simulink model "vegas01_4tap_1024ch_220_r8b_opt"  (Simulink 7.5) (continued)
== system vegas01_4tap_1024ch_220_r8b_opt == (continued)
      | input_bit_width = eval(get_param(gcb,'input_bit_width'));
      | if input_bit_width + bit_growth_sum > 18
      |     disp('Total bit width cannot exceed 18. Forcing to 0 bit growth');
      |     set_param(gcb,'bit_growth_chart','[0]');
      | end|||||||||||fft_callback_arch(gcb);||||specify_mult = get_param(gcb, 'specify_mult');
      | set_param_state(gcb, 'mult_spec', specify_mult);||hardcode_shifts = get_param(gcb, 'hardcode_shifts');
      | set_param_state(gcb, 'shift_schedule', hardcode_shifts);||fft_callback_dsp48_adders(gcb);|bit_growth_chart = eval(get_param(gcb,'bit_growth_chart'));
      | FFTSize = eval(get_param(gcb,'FFTSize'));
      | bit_growth_chart = [reshape(bit_growth_chart, 1, []) zeros(1,FFTSize)];
      | bit_growth_sum = sum(bit_growth_chart(1:FFTSize));
      | input_bit_width = eval(get_param(gcb,'input_bit_width'));
      | if input_bit_width + bit_growth_sum > 18
      |     disp('Total bit width cannot exceed 18. Forcing to 0 bit growth');
      |     set_param(gcb,'bit_growth_chart','[0]');
      | end||
      mask TabNameString: Basic,Basic,Basic,Basic,Basic,Latency,Latency,Latency,Latency,Latency,Latency,Implementation,Implementation,Implementation,Implementation,Implementation,Implementation,Implementation,Implementation,Implementation,Implementation,Implementation,Basic,Basic,Basic
  gbe0 : xps_library/ten_Gbe_v2  Tag=xps:tengbe_v2  SystemSampleTime=-1  port=0  rx_dist_ram=on  large_frames=on  show_param=on  pre_emph=3  swing=800  fab_en=off  fab_mac=hex2dec('123456780000')  fab_ip=10*(2^24) + 0*(2^16) + 0*(2^8) + 140*(2^0)  fab_udp=60001  fab_gate=1  cpu_rx_en=on  cpu_tx_en=on
  gpio : xps_library/gpio  Tag=xps:gpio  SystemSampleTime=-1  io_group=ROACH:gpiob  io_dir=in  arith_type=Unsigned  bitwidth=4  bin_pt=0  bit_index=[1:4]  sample_period=1  use_single_ended=off  use_ddr=off  reg_iob=on  reg_clk_phase=0  termination=None
      link override ssg_speader_r2_gpio_gateway: arith_type=Unsigned  n_bits=4  bin_pt=0  period=sample_period
  gpio_tig_reg_1 : xbsIndex_r4/Register  init=0  rst=off  en=off  dbl_ovrd=off  block_type=register
      settings: block_version=11.4
  gpio_tig_reg_2 : xbsIndex_r4/Register  init=0  rst=off  en=off  dbl_ovrd=off  block_type=register
      settings: block_version=11.4
  gpio_tig_reg_3 : xbsIndex_r4/Register  init=0  rst=off  en=off  dbl_ovrd=off  block_type=register
      settings: block_version=11.4
  leds_gpios : Subsystem
  length : xps_library/software register  Tag=xps:sw_reg  SystemSampleTime=-1  io_dir=From Processor  arith_type=Unsigned  bitwidth=32  bin_pt=0  sample_period=1  gw_name=vegas01_4tap_1024ch_220_r8b_length_user_data_out
      link override ssg_speader_r2_length_user_data_out: arith_type=Unsigned  n_bits=32  bin_pt=0  period=1
  manrst : xps_library/software register  Tag=xps:sw_reg  SystemSampleTime=-1  io_dir=From Processor  arith_type=Unsigned  bitwidth=32  bin_pt=0  sample_period=1  gw_name=vegas01_4tap_1024ch_220_r8b_manrst_user_data_out
      link override convert: arith_type=Unsigned  n_bits=32  bin_pt=0
      link override r_spec_2048_r102_cnt_rst_user_data_out: arith_type=Unsigned  n_bits=32  bin_pt=0  period=1
      link override r_spec_2048_r102_nosim_cnt_rst_user_data_out: arith_type=Unsigned  n_bits=32  bin_pt=0  period=1
      link override r_spec_2048_r103_cnt_rst_user_data_out: arith_type=Unsigned  n_bits=32  bin_pt=0  period=1
      link override roach_spec_no10gbe_sync_cnt_rst_user_data_out: arith_type=Unsigned  n_bits=32  bin_pt=0  period=1
      link override roach_spec_rst_cnt_user_data_out: arith_type=Unsigned  n_bits=32  bin_pt=0  period=1
      link override roach_spec_rst_sync_cnt_user_data_out: arith_type=Unsigned  n_bits=32  bin_pt=0  period=1
  master_slave_sel : xps_library/software register  Tag=xps:sw_reg  SystemSampleTime=-1  io_dir=From Processor  arith_type=Unsigned  bitwidth=32  bin_pt=0  sample_period=1  gw_name=vegas01_4tap_1024ch_220_r8b_master_slave_sel_user_data_out
      link override ssg_speader_r2_master_slave_sel_user_data_out: arith_type=Unsigned  n_bits=32  bin_pt=0  period=1
  negedge : casper_library_misc/negedge  Tag=Rising Edge Detector  SystemSampleTime=-1
  negedge1 : casper_library_misc/negedge  Tag=Rising Edge Detector  SystemSampleTime=-1
  negedge2 : casper_library_misc/negedge  Tag=Rising Edge Detector  SystemSampleTime=-1
  pfb_fir_real1 : Subsystem  mask(pfb_coeff_gen_dual){pfb_size=11, n_sim_inputs=3, n_taps=4, data_n_bits=8, coeff_n_bits=18, output_bit_width=18, window_fn=hamming, bin_width=1, end_scale_val=1, endDelay=on, cheap_sync=off, autoplace_mode='Attached to ADC0', autoplace_optimize=off, bram_latency_coeff=off, bram_latency_delay=off, register_counter=on, pack_delays=on}
      mask prompts: pfb_size "PFB Size (2^?)"; n_sim_inputs "# Simultaneous Inputs (2^?); Max=6"; n_taps "# Taps"; data_n_bits "Input bit Width"; coeff_n_bits "# Coefficient Bits"; output_bit_width "Output bit width"; window_fn "Window Function"; bin_width "Channel Width"; end_scale_val "Downscale at end (2^?)"; endDelay "Delay at end?"; cheap_sync "Use cheap Sync hardware?"; autoplace_mode "Autoplacement Mode?"; autoplace_optimize "Optimize parameters for auto-placement? (settings below here ignored)"; bram_latency_coeff "Register Coefficient Brams?"; bram_latency_delay "Register Delay Brams?"; register_counter "Register Delay Counters? (0 or 1)"; pack_delays "Pack delays cross-input?"
      mask init:
      | global drawing_parameters;
      | % drawing_parameters.pfb_size=pfb_size;
      | % drawing_parameters.n_sim_inputs=n_sim_inputs;
      | % drawing_parameters.n_taps=n_taps;
      | % drawing_parameters.data_n_bits=data_n_bits;
      | % drawing_parameters.data_bin_pt=data_bin_pt;
      | % drawing_parameters.coeff_n_bits=coeff_n_bits;
      | % drawing_parameters.coeff_bin_pt=coeff_n_bits-1;
      | % drawing_parameters.window_fn=window_fn;
      | % drawing_parameters.mem_type=mem_type;
      | % drawing_parameters.bram_latency=bram_latency;
      | % drawing_parameters.bin_width=bin_width;
      | % drawing_parameters.end_scale_val=end_scale_val;
      | % drawing_parameters.cheap_sync=cheap_sync;
      | config.source=str2func('pfb_improved_draw');
      | config.toplevel=gcb;
      | config.debug=0;
      | config.depend={'pfb_improved_draw.m','pfb_coeff_gen_dual_draw.m'};
      | xBlock(config,{pfb_size,n_sim_inputs,n_taps,data_n_bits,coeff_n_bits, ...
      |                output_bit_width,window_fn,bram_latency_coeff+2,bram_latency_delay+2,bin_width,end_scale_val,endDelay, cheap_sync,register_counter, pack_delays, autoplace_mode, autoplace_optimize});
      mask StyleString: edit,edit,edit,edit,edit,edit,popup(bartlett|barthannwin|blackman|blackmanharris|bohamwin|chebwin|flattopwin|gausswin|hamming|hann|kaiser|nuttallwin|parzenwin|rectwin|tukeywin|triang),edit,edit,checkbox,checkbox,popup('Attached to ADC0'|'Attached to ADC1'|'Attached to both ADC0 and ADC1 (interleaved)'),checkbox,checkbox,checkbox,checkbox,checkbox
  pfb_fir_real3 : Subsystem  mask(pfb_coeff_gen_dual){pfb_size=11, n_sim_inputs=3, n_taps=4, data_n_bits=8, coeff_n_bits=18, output_bit_width=18, window_fn=hamming, bin_width=1, end_scale_val=1, endDelay=on, cheap_sync=off, autoplace_mode='Attached to ADC0', autoplace_optimize=off, bram_latency_coeff=off, bram_latency_delay=off, register_counter=on, pack_delays=on}
      mask prompts: pfb_size "PFB Size (2^?)"; n_sim_inputs "# Simultaneous Inputs (2^?); Max=6"; n_taps "# Taps"; data_n_bits "Input bit Width"; coeff_n_bits "# Coefficient Bits"; output_bit_width "Output bit width"; window_fn "Window Function"; bin_width "Channel Width"; end_scale_val "Downscale at end (2^?)"; endDelay "Delay at end?"; cheap_sync "Use cheap Sync hardware?"; autoplace_mode "Autoplacement Mode?"; autoplace_optimize "Optimize parameters for auto-placement? (settings below here ignored)"; bram_latency_coeff "Register Coefficient Brams?"; bram_latency_delay "Register Delay Brams?"; register_counter "Register Delay Counters? (0 or 1)"; pack_delays "Pack delays cross-input?"
      mask init: same as vegas01_4tap_1024ch_220_r8b_opt/pfb_fir_real1
      mask StyleString: edit,edit,edit,edit,edit,edit,popup(bartlett|barthannwin|blackman|blackmanharris|bohamwin|chebwin|flattopwin|gausswin|hamming|hann|kaiser|nuttallwin|parzenwin|rectwin|tukeywin|triang),edit,edit,checkbox,checkbox,popup('Attached to ADC0'|'Attached to ADC1'|'Attached to both ADC0 and ADC1 (interleaved)'),checkbox,checkbox,checkbox,checkbox,checkbox
  posedge1 : casper_library_misc/posedge  Tag=Rising Edge Detector  SystemSampleTime=-1
  posedge3 : casper_library_misc/posedge  Tag=Rising Edge Detector  SystemSampleTime=-1
  posedge7 : casper_library_misc/posedge  Tag=Rising Edge Detector  SystemSampleTime=-1
  reinterp_vacc{,1..15} (16 alike) : xbsIndex_r4/Reinterpret  force_arith_type=on  arith_type="Signed  (2's comp)"  force_bin_pt=off  bin_pt=0  block_type=reinterpret
  reshaper : Subsystem  mask{inwid=512, inlen=256, outwid=64, outchunk=1024, headlen=2000}
      mask prompts: inwid "Input Width (bits)"; inlen "Input Length (clocks)"; outwid "Output Width (bits)"; outchunk "Output Chunk Length (clocks)"; headlen "Header Length"
      mask help:
      | This block takes wide input data and reshapes it to a narrower (or the same)
      | bit width, and breaks it into packets, between which a header can be inserted.
      | Each input data frame must be proceeded by a reset pulse. The input data can be
      | disjoint, with a pulsed valid signal. When the reset signal is pulsed, the previous data
      | frame will be read out. Therefore the number of clocks to read out the header and
      | data must be greater than the number of clocks between two frames (rst signals).
      |
      | The sel signal can be used to drive the select input of a mux which muxes between
      | the data stream (dout) and the indicated number of header words. The header_valid_out
      | signal goes high for each word of the header. dout should be connected to input
      | 0 of the mux. The data_valid_out goes high for each output data value. The st output
      | is for debug access to the internal state machine state.
      mask init:
      | l2inlen = ceil(log2(inlen))
      | l2outchunk = ceil(log2(outchunk))
      | outlen = inlen*(inwid/outwid)
      | nchunk = outlen/outchunk
      | l2headlen = ceil(log2(headlen))
      | l2outlen = ceil(log2(outlen))
  sg : Subsystem
  speader1 : Subsystem  AncestorBlock=gbt_library/speader  mask{spead_msw=64, spead_lsw=40, num_items=6, num_payloads=2}
      mask prompts: num_items "Number of items (+5)"; num_payloads "number of payloads"
      mask help:
      | The size of the heap and payload are in Bytes.
      | If there is one packet per heap then payload_offset is 0.
      | All the input must be not longer than 40 bits.
  spectrum_cntr : xbsIndex_r4/Counter  cnt_type=Free Running  cnt_to=Inf  operation=Up  start_count=0  cnt_by_val=1  arith_type=Unsigned  n_bits=40  bin_pt=0  load_pin=off  rst=on  en=on  explicit_period=on  period=1  dbl_ovrd=off  use_behavioral_HDL=off  implementation=Fabric  block_type=counter
      settings: block_version=11.4
  ssg_bram : Subsystem  Tag=xps:bram  AncestorBlock=xps_library/Shared BRAM  mask{arith_type=Unsigned, addr_width=10, data_width=32, data_bin_pt=0, init_vals=[12801,121600,3201,3203,57602,3203,3201,121600,12801,12805,121604,3205,3207,57606,3207,3205,121604,12805,0:0], sample_rate=1}
      mask prompts: arith_type "Output Data Type"; addr_width "Address width"; data_width "Data Width"; data_bin_pt "Data Binary Point"; init_vals "Initial 32-bit values (simulation only)"; sample_rate "Sample rate"
      mask init:
      | shared_bram_mask;
      mask StyleString: popup(Unsigned|Signed  (2's comp)),edit,popup(8|16|32|64|128),edit,edit,edit
  ssg_bram_delay1 : xbsIndex_r4/Delay  rst=off  infoeditControl=Selection of Reset will increase slice count due to use of real FFs and instead of SRLs  en=off  latency=1  dbl_ovrd=off  reg_retiming=on  block_type=delay
  ssg_bram_delay2 : xbsIndex_r4/Delay  rst=off  infoeditControl=Selection of Reset will increase slice count due to use of real FFs and instead of SRLs  en=off  latency=1  dbl_ovrd=off  reg_retiming=off  block_type=delay
  stokes : Subsystem  mask{n_samples=4, addlatency=2}
      mask prompts: n_samples "Number of Simultaneous Inputs"; addlatency "Add Latency"
      mask init:
      | % % To make the xBlock smart so it won''t re-generate the
      | % % subsystem if neither the arguments nor the scripts are
      | % % changes, use as the following:
      | % cfg.source = str2func('stokes_init');
      | % cfg.toplevel = gcb;
      | % cfg.depend = {'stokes_init'};
      | % args = {n_samples, addlatency};
      | % xBlock(cfg, args);
      mask TabNameString: Basic,Basic
  time_cntr : xbsIndex_r4/Counter  cnt_type=Free Running  cnt_to=Inf  operation=Up  start_count=0  cnt_by_val=1  arith_type=Unsigned  n_bits=40  bin_pt=0  load_pin=off  rst=on  en=off  explicit_period=on  period=1  dbl_ovrd=off  use_behavioral_HDL=off  implementation=Fabric  block_type=counter
      settings: block_version=11.4
  top bit : xbsIndex_r4/Slice  nbits=1  boolean_output=on  mode=Upper Bit Location + Width  bit1=0  base1=MSB of Input  bit0=0  base0=LSB of Input  dbl_ovrd=off  block_type=slice
  vacc0 : Subsystem  AncestorBlock=gavrt_library/vacc  mask(vacc){veclen=8, n_inputs=4, max_accum=10, arith_type=1, in_bit_width=32, in_bin_pt=0, out_bit_width=42, out_bin_pt=0, register_din=1, add_latency=2, bram_latency=2, mux_latency=1, use_dsp48=1}
      mask prompts: veclen "Vector Length (2^?)"; n_inputs "Number of Simultaneous Vectors"; max_accum "Maximum # Accumulations (2^?)"; arith_type "Arithmetic Type (0=Unsigned, 1=Signed)"; in_bit_width "Input Bit Width"; in_bin_pt "Input Binary Point"; out_bit_width "Output Bit Width"; out_bin_pt "Output Binary Point"; register_din "Register Input Data  (1 = Enable Register Retiming on input delay)"; add_latency "Add Latency"; bram_latency "BRAM Latency"; mux_latency "Mux Latency"; use_dsp48 "Use DSP48"
      mask help:
      | Sync is expected the clock before the first data to be accumulated.
      | Use the Cram block to feed din for multiple simultaneous inputs.
      | acc_len is the number of vectors to sum.
      | valid is high while a valid accumulation is being clocked out on dout.
      | If only one sync pulse is provided, valid accumulations will repeatedly appear on dout as they become available.
      | Undesired behavior may occur if acc_len is changed without a new sync pulse.
      | Note: The output is an unsigned concatenation of the output streams. Thus, even with only one stream, the output must be recast before using.
      | Register Input Data controls the "Enable Register Retiming" parameter of the delays on 'din'.
      mask init:
      | config.toplevel = gcb;
      | config.source = str2func('vacc_init_xblock');
      | xBlock( config, {...
      |     'veclen', veclen, ...
      |     'n_inputs', n_inputs, ...
      |     'max_accum', max_accum, ...
      |     'arith_type', arith_type, ...
      |     'in_bit_width', in_bit_width, ...
      |     'in_bin_pt', in_bin_pt, ...
      |     'out_bit_width', out_bit_width, ...
      |     'out_bin_pt', out_bin_pt, ...
      |     'register_din', register_din, ...
      |     'add_latency', add_latency, ...
      |     'bram_latency', bram_latency, ...
      |     'mux_latency', mux_latency, ...
      |     'use_dsp48', use_dsp48, ...
      | });
      settings: AttributesFormatString=|
      | vector length=32, inputs=4
      | max accumulations=2^5
  vacc1 : Subsystem  AncestorBlock=gavrt_library/vacc  mask(vacc){veclen=8, n_inputs=4, max_accum=10, arith_type=1, in_bit_width=32, in_bin_pt=0, out_bit_width=42, out_bin_pt=0, register_din=1, add_latency=2, bram_latency=2, mux_latency=1, use_dsp48=1}
      mask prompts: veclen "Vector Length (2^?)"; n_inputs "Number of Simultaneous Vectors"; max_accum "Maximum # Accumulations (2^?)"; arith_type "Arithmetic Type (0=Unsigned, 1=Signed)"; in_bit_width "Input Bit Width"; in_bin_pt "Input Binary Point"; out_bit_width "Output Bit Width"; out_bin_pt "Output Binary Point"; register_din "Register Input Data  (1 = Enable Register Retiming on input delay)"; add_latency "Add Latency"; bram_latency "BRAM Latency"; mux_latency "Mux Latency"; use_dsp48 "Use DSP48"
      mask help:
      | Sync is expected the clock before the first data to be accumulated.
      | Use the Cram block to feed din for multiple simultaneous inputs.
      | acc_len is the number of vectors to sum.
      | valid is high while a valid accumulation is being clocked out on dout.
      | If only one sync pulse is provided, valid accumulations will repeatedly appear on dout as they become available.
      | Undesired behavior may occur if acc_len is changed without a new sync pulse.
      | Note: The output is an unsigned concatenation of the output streams. Thus, even with only one stream, the output must be recast before using.
      | Register Input Data controls the "Enable Register Retiming" parameter of the delays on 'din'.
      mask init: same as vegas01_4tap_1024ch_220_r8b_opt/vacc0
      settings: AttributesFormatString=|
      | vector length=32, inputs=4
      | max accumulations=2^5
  vacc2 : Subsystem  AncestorBlock=gavrt_library/vacc  mask(vacc){veclen=8, n_inputs=4, max_accum=10, arith_type=1, in_bit_width=32, in_bin_pt=0, out_bit_width=42, out_bin_pt=0, register_din=1, add_latency=2, bram_latency=2, mux_latency=1, use_dsp48=1}
      mask prompts: veclen "Vector Length (2^?)"; n_inputs "Number of Simultaneous Vectors"; max_accum "Maximum # Accumulations (2^?)"; arith_type "Arithmetic Type (0=Unsigned, 1=Signed)"; in_bit_width "Input Bit Width"; in_bin_pt "Input Binary Point"; out_bit_width "Output Bit Width"; out_bin_pt "Output Binary Point"; register_din "Register Input Data  (1 = Enable Register Retiming on input delay)"; add_latency "Add Latency"; bram_latency "BRAM Latency"; mux_latency "Mux Latency"; use_dsp48 "Use DSP48"
      mask help:
      | Sync is expected the clock before the first data to be accumulated.
      | Use the Cram block to feed din for multiple simultaneous inputs.
      | acc_len is the number of vectors to sum.
      | valid is high while a valid accumulation is being clocked out on dout.
      | If only one sync pulse is provided, valid accumulations will repeatedly appear on dout as they become available.
      | Undesired behavior may occur if acc_len is changed without a new sync pulse.
      | Note: The output is an unsigned concatenation of the output streams. Thus, even with only one stream, the output must be recast before using.
      | Register Input Data controls the "Enable Register Retiming" parameter of the delays on 'din'.
      mask init: same as vegas01_4tap_1024ch_220_r8b_opt/vacc0
      settings: AttributesFormatString=|
      | vector length=32, inputs=4
      | max accumulations=2^5
  vacc3 : Subsystem  AncestorBlock=gavrt_library/vacc  mask(vacc){veclen=8, n_inputs=4, max_accum=10, arith_type=1, in_bit_width=32, in_bin_pt=0, out_bit_width=42, out_bin_pt=0, register_din=1, add_latency=2, bram_latency=2, mux_latency=1, use_dsp48=1}
      mask prompts: veclen "Vector Length (2^?)"; n_inputs "Number of Simultaneous Vectors"; max_accum "Maximum # Accumulations (2^?)"; arith_type "Arithmetic Type (0=Unsigned, 1=Signed)"; in_bit_width "Input Bit Width"; in_bin_pt "Input Binary Point"; out_bit_width "Output Bit Width"; out_bin_pt "Output Binary Point"; register_din "Register Input Data  (1 = Enable Register Retiming on input delay)"; add_latency "Add Latency"; bram_latency "BRAM Latency"; mux_latency "Mux Latency"; use_dsp48 "Use DSP48"
      mask help:
      | Sync is expected the clock before the first data to be accumulated.
      | Use the Cram block to feed din for multiple simultaneous inputs.
      | acc_len is the number of vectors to sum.
      | valid is high while a valid accumulation is being clocked out on dout.
      | If only one sync pulse is provided, valid accumulations will repeatedly appear on dout as they become available.
      | Undesired behavior may occur if acc_len is changed without a new sync pulse.
      | Note: The output is an unsigned concatenation of the output streams. Thus, even with only one stream, the output must be recast before using.
      | Register Input Data controls the "Enable Register Retiming" parameter of the delays on 'din'.
      mask init: same as vegas01_4tap_1024ch_220_r8b_opt/vacc0
      settings: AttributesFormatString=|
      | vector length=32, inputs=4
      | max accumulations=2^5
  wires:
  manrst/1 -> Register16/1
  Constant19/1 -> manrst/1
  Register16/1 -> Slice2/1, Slice4/1
  Slice2/1 -> Delay26/1
  Register10/1 -> [xout0]
  Register11/1 -> [xout1]
  Register9/1 -> Delay2/1
  Register48/1 -> [yout0]
  Register49/1 -> [yout1]
  Constant4/1 -> adc083000x2/4(adc1_data_valid_sim), adc083000x2/3(adc1_sync_sim), adc083000x2/2(adc0_data_valid_sim)
  Constant1/1 -> adc083000x2/1(adc0_sync_sim)
  Slice10/1 -> [adc_sync]
  Register44/1 -> Slice10/1
  adc083000x2/1(adc0_s0) -> Delay7/1
  adc083000x2/9(adc0_sync) -> Register44/1
  adc083000x2/10(adc0_outofrange) -> Terminator44/1
  adc083000x2/11(adc0_data_valid) -> Terminator45/1
  adc083000x2/12(adc1_s0) -> Delay27/1
  adc083000x2/20(adc1_sync) -> Terminator43/1
  adc083000x2/21(adc1_outofrange) -> Terminator46/1
  adc083000x2/22(adc1_data_valid) -> Terminator47/1
  Constant20/1 -> adc083000x2/5(sim_adc0_data_in)
  Constant21/1 -> adc083000x2/6(sim_adc1_data_in)
  Concat1/1 -> [default_ip]
  Constant6/1 -> gbe0/7
  gbe0/10 -> Terminator/1
  gbe0/2 -> Terminator17/1
  gbe0/3 -> Terminator16/1
  gbe0/4 -> Terminator15/1
  gbe0/5 -> [txack]
  gbe0/6 -> Terminator8/1
  gbe0/7 -> Terminator6/1
  gbe0/8 -> Terminator5/1
  gbe0/9 -> Terminator3/1
  gbe0/11 -> Terminator11/1
  Constant5/1 -> gbe0/8
  Delay21/1 -> gbe0/6
  Delay25/1 -> gbe0/3
  Delay29/1 -> gbe0/2
  Delay30/1 -> gbe0/1
  acc_len/1 -> Delay32/1
  Constant3/1 -> acc_len/1
  Delay32/1 -> [acc_len]
  Constant2/1 -> [fftshift]
  adc083000x2/16(adc1_s4) -> Delay34/1
  adc083000x2/13(adc1_s1) -> Delay28/1
  adc083000x2/15(adc1_s3) -> Delay33/1
  adc083000x2/17(adc1_s5) -> Delay35/1
  adc083000x2/19(adc1_s7) -> Delay37/1
  adc083000x2/3(adc0_s2) -> Delay18/1
  adc083000x2/5(adc0_s4) -> Delay20/1
  adc083000x2/7(adc0_s6) -> Delay23/1
  adc083000x2/2(adc0_s1) -> Delay9/1
  adc083000x2/4(adc0_s3) -> Delay19/1
  adc083000x2/6(adc0_s5) -> Delay22/1
  adc083000x2/8(adc0_s7) -> Delay24/1
  adc083000x2/14(adc1_s2) -> Delay31/1
  adc083000x2/18(adc1_s6) -> Delay36/1
  err_clear_sim1/1 -> dest_ip/1
  dest_ip/1 -> [dest_ip]
  dest_port/1 -> Slice7/1
  err_clear_sim3/1 -> dest_port/1
  Slice7/1 -> [dest_port]
  Constant/1 -> Concat1/1
  Constant7/1 -> Concat1/2
  Constant8/1 -> Concat1/3
  Constant9/1 -> Concat1/4
  [tge_rst] -> Delay30/1
  [data_out] -> speader1/1(data_in)
  [data_valid] -> speader1/2(valid_in)
  [dest_ip] -> gbe0/4
  [dest_port] -> gbe0/5
  speader1/3(eof) -> [eof]
  [eof] -> Delay21/1
  Constant18/1 -> speader1/3(heap_size)
  Constant33/1 -> speader1/8(mode)
  Constant34/1 -> speader1/10(payload_offset)
  speader1/2(data_valid) -> [data_valid_spead]
  [data_valid_spead] -> Delay25/1
  [data_spead] -> Delay29/1
  [master_rst1] -> time_cntr/1
  Slice5/1 -> [xxout0]
  Slice6/1 -> [yyout0]
  Slice13/1 -> [xyoutre0]
  Slice18/1 -> [xyoutim0]
  Slice19/1 -> [xxout1]
  Slice20/1 -> [yyout1]
  Slice21/1 -> [xyoutre1]
  Slice22/1 -> [xyoutim1]
  [acc_len] -> Convert/1
  Constant24/1 -> speader1/4(Packet_length)
  [master_rst1] -> spectrum_cntr/1
  [eoh] -> spectrum_cntr/2
  [spead_rst] -> speader1/11(Reset)
  Convert/1 -> speader1/7(integration size)
  speader1/1(data_out) -> [data_spead]
  fft_wideband_real1/1(sync_out) -> Register9/1
  [sync_gen_1] -> pfb_fir_real1/1(sync)
  [sync_gen_0] -> pfb_fir_real3/1(sync)
  [a0] -> pfb_fir_real1/2(in0)
  [a1] -> pfb_fir_real1/3(in1)
  [a2] -> pfb_fir_real1/4(in2)
  [a3] -> pfb_fir_real1/5(in3)
  [a4] -> pfb_fir_real1/6(in4)
  [a5] -> pfb_fir_real1/7(in5)
  [a6] -> pfb_fir_real1/8(in6)
  [a7] -> pfb_fir_real1/9(in7)
  [b0] -> pfb_fir_real3/2(in0)
  [b1] -> pfb_fir_real3/3(in1)
  [b2] -> pfb_fir_real3/4(in2)
  [b3] -> pfb_fir_real3/5(in3)
  [b4] -> pfb_fir_real3/6(in4)
  [b5] -> pfb_fir_real3/7(in5)
  [b6] -> pfb_fir_real3/8(in6)
  [b7] -> pfb_fir_real3/9(in7)
  [fftshift] -> Delay5/1
  Delay6/1 -> fft_wideband_real/2(shift)
  Register1/1 -> [xout2]
  Register2/1 -> [xout3]
  fft_wideband_real/1(sync_out) -> Terminator2/1
  Register12/1 -> [yout2]
  Register13/1 -> [yout3]
  [xout0] -> stokes/1(x0)
  [yout0] -> stokes/2(y0)
  [xout1] -> stokes/3(x1)
  [yout1] -> stokes/4(y1)
  [xout2] -> stokes/5(x2)
  [yout2] -> stokes/6(y2)
  [xout3] -> stokes/7(x3)
  [yout3] -> stokes/8(y3)
  Slice29/1 -> [xxout2]
  Slice30/1 -> [yyout2]
  Slice14/1 -> [xyoutre2]
  Slice15/1 -> [xyoutim2]
  Slice25/1 -> [xxout3]
  Slice26/1 -> [yyout3]
  Slice27/1 -> [xyoutre3]
  Slice28/1 -> [xyoutim3]
  stokes/1(x0x0*) -> Slice5/1
  stokes/2(y0y0*) -> Slice6/1
  stokes/3(x0y0*_re) -> Slice13/1
  stokes/4(x0y0*_im) -> Slice18/1
  stokes/5(x1x1*) -> Slice19/1
  stokes/6(y1y1*) -> Slice20/1
  stokes/7(x1y1*_re) -> Slice21/1
  stokes/8(x1y1*_im) -> Slice22/1
  stokes/9(x2x2*) -> Slice29/1
  stokes/10(y2y2*) -> Slice30/1
  stokes/11(x2y2*_re) -> Slice14/1
  stokes/12(x2y2*_im) -> Slice15/1
  stokes/13(x3x3*) -> Slice25/1
  stokes/14(y3y3*) -> Slice26/1
  stokes/15(x3y3*_re) -> Slice27/1
  stokes/16(x3y3*_im) -> Slice28/1
  spectrum_cntr/1 -> [spec_cntr]
  time_cntr/1 -> [time_cntr]
  [time_cntr] -> speader1/5(time_stamp)
  [spec_cntr] -> speader1/6(spectrum counter)
  cram4/1(Out) -> [din]
  speader1/4(eoh) -> [eoh]
  pfb_fir_real3/2(out0) -> fft_wideband_real/3(din_0)
  pfb_fir_real3/3(out1) -> fft_wideband_real/4(din_1)
  pfb_fir_real3/4(out2) -> fft_wideband_real/5(din_2)
  pfb_fir_real3/5(out3) -> fft_wideband_real/6(din_3)
  pfb_fir_real3/6(out4) -> fft_wideband_real/7(din_4)
  pfb_fir_real3/7(out5) -> fft_wideband_real/8(din_5)
  pfb_fir_real3/8(out6) -> fft_wideband_real/9(din_6)
  pfb_fir_real3/9(out7) -> fft_wideband_real/10(din_7)
  fft_wideband_real/5(dout3) -> Register13/1
  fft_wideband_real/3(dout1) -> Register49/1
  fft_wideband_real/2(dout0) -> Register48/1
  fft_wideband_real/4(dout2) -> Register12/1
  fft_wideband_real/6(of) -> Terminator30/1
  pfb_fir_real3/1(sync_out) -> fft_wideband_real/1(sync)
  pfb_fir_real1/1(sync_out) -> fft_wideband_real1/1(sync)
  fft_wideband_real1/2(dout0) -> Register10/1
  fft_wideband_real1/3(dout1) -> Register11/1
  fft_wideband_real1/6(of) -> Terminator12/1
  reshaper/2(sel) -> Terminator10/1
  reshaper/3(header_valid_out) -> Terminator13/1
  [din] -> Delay13/1
  Delay10/1 -> Delay11/1, negedge2/1
  Delay13/1 -> Delay12/1
  Delay12/1 -> reshaper/3(din)
  Delay11/1 -> reshaper/2(valid)
  reshaper/5(st) -> [reshape_state]
  Delay14/1 -> [data_out]
  Delay15/1 -> [data_valid]
  reshaper/1(dout) -> Delay14/1
  reshaper/4(data_valid_out) -> Delay15/1
  Constant27/1 -> ssg_bram/2(data_in)
  Constant28/1 -> ssg_bram/3(we)
  Counter/1 -> ssg_bram/1(addr)
  ssg_bram/1(data_out) -> ssg_bram_delay1/1
  SuperSlice/1(DUR) -> [DUR]
  SuperSlice/2(AS/R) -> [ASR]
  SuperSlice/3(S/R1) -> [SR1]
  SuperSlice/4(S/R0) -> [SR0]
  SuperSlice/5(CAL) -> [CAL]
  SuperSlice/6(BLANK) -> [LOC_BLANK]
  Constant30/1 -> Register19/1
  Register18/1 -> Inverter/1
  Inverter/1 -> Register19/2, [spead_rst]  "ppsready"
  Constant31/1 -> Register18/1
  arm/1 -> Register20/1
  Constant12/1 -> arm/1
  Register20/1 -> Slice3/1
  Slice3/1 -> [arm]
  [arm] -> Register18/2
  [master_rst2] -> Register18/3
  [sync_out] -> Register19/3
  Register19/1 -> [data_enable]
  [valid] -> Logical/1
  [data_enable] -> Logical/2
  Logical/1 -> Delay10/1, negedge1/1
  Delay4/1 -> speader1/9(status_bits)
  Delay5/1 -> fft_wideband_real1/2(shift)
  [fftshift] -> Delay6/1
  [adc_sync] -> sg/1(pps)
  gbe0/1 -> Terminator9/1
  negedge2/1 -> reshaper/1(rst)
  Slice4/1 -> [auto_reset_enable]
  [arm] -> posedge3/1
  posedge3/1 -> Logical2/2
  Logical2/1 -> [tge_rst]
  Delay26/1 -> Logical2/1
  Logical3/1 -> Logical2/3
  [auto_reset_enable] -> Logical3/1
  [txack] -> posedge1/1
  posedge1/1 -> Logical3/2
  Constant11/1 -> length/1
  length/1 -> cycle_limit/2(cnt_limit)
  dur_len/1(at_limit) -> Counter/2
  cycle_limit/1(at_limit) -> Counter/1
  [spec_tick] -> cycle_limit/1(cnt_en), dur_len/1(cnt_en)
  [DUR] -> dur_len/2(cnt_limit)
  [master_rst3] -> dur_len/3(master_rst)
  [master_rst3] -> cycle_limit/3(master_rst)
  [SR0] -> bus_create4/3(in3)
  [CAL] -> bus_create4/2(in2)
  [LOC_BLANK] -> bus_create4/1(in1)
  bus_create4/1(bus_out) -> gpio_tig_reg_2/1
  bus_expand3/2(out3) -> leds_gpios/1(IN0)
  bus_expand3/3(out2) -> leds_gpios/2(IN1)
  bus_expand3/4(lsb_out1) -> leds_gpios/3(IN2)
  err_clear_sim5/1 -> gpio/1
  gpio/1 -> gpio_tig_reg_1/1
  [spec_tick] -> Register22/2
  err_clear_sim6/1 -> master_slave_sel/1
  bit 0 and 1/1 -> Mux3/1
  master_slave_sel/1 -> bit 0 and 1/1, bit 2/1
  Mux2/1 -> gpio_tig_reg_3/1
  bit 2/1 -> [ssg_bits_gpios]
  [ssg_bits_gpios] -> Mux2/1
  Register22/1 -> Mux3/3, Mux2/3
  gpio_tig_reg_2/1 -> Mux3/2, Mux2/2
  Mux3/1 -> top bit/1, Register25/1
  top bit/1 -> [blank]
  [int_tick] -> Register25/2
  Register25/1 -> [status_bits]
  [master_rst4] -> Counter1/1
  Counter1/1 -> Slice8/1, Slice9/1
  Slice8/1 -> negedge/1
  Slice9/1 -> [spec_cnt]
  negedge/1 -> [spec_tick]
  negedge1/1 -> [int_tick]
  Constant10/1 -> bus_create2/1(in1)
  [status_bits] -> bus_create2/2(in2)
  bus_create2/1(bus_out) -> Delay4/1
  posedge7/1 -> [anew_acc]
  vacc0/2(dout_1_1) -> Slice1/1
  vacc0/3(dout_2_1) -> Slice11/1
  vacc1/2(dout_1_1) -> Slice17/1
  vacc1/3(dout_2_1) -> Slice23/1
  vacc2/2(dout_1_1) -> Slice32/1
  vacc2/3(dout_2_1) -> Slice33/1
  vacc3/2(dout_1_1) -> Slice36/1
  vacc3/3(dout_2_1) -> Slice37/1
  vacc0/4(dout_3_1) -> Slice12/1
  vacc0/5(dout_4_1) -> Slice16/1
  vacc2/4(dout_3_1) -> Slice34/1
  vacc2/5(dout_4_1) -> Slice35/1
  vacc3/4(dout_3_1) -> Slice38/1
  vacc3/5(dout_4_1) -> Slice39/1
  [sync_out0] -> vacc0/1(sync)
  [acc_len] -> vacc0/2(acc_len)
  [acc_len] -> vacc3/2(acc_len)
  [sync_out2] -> vacc2/1(sync)
  [sync_out3] -> vacc3/1(sync)
  [acc_len] -> vacc2/2(acc_len)
  vacc1/1(valid) -> [valid]
  vacc2/1(valid) -> Terminator4/1
  vacc3/1(valid) -> posedge7/1, Terminator7/1
  vacc1/4(dout_3_1) -> Slice24/1
  vacc1/5(dout_4_1) -> Slice31/1
  [sync_out1] -> vacc1/1(sync)
  [acc_len] -> vacc1/2(acc_len)
  [xxout0] -> reinterp_vacc/1
  [yyout0] -> reinterp_vacc1/1
  [xyoutre0] -> reinterp_vacc2/1
  [xyoutim0] -> reinterp_vacc3/1
  [xxout1] -> reinterp_vacc4/1
  [yyout1] -> reinterp_vacc5/1
  [xyoutre1] -> reinterp_vacc6/1
  [xyoutim1] -> reinterp_vacc7/1
  [xxout2] -> reinterp_vacc8/1
  [yyout2] -> reinterp_vacc9/1
  [xyoutre2] -> reinterp_vacc10/1
  [xyoutim2] -> reinterp_vacc11/1
  [xxout3] -> reinterp_vacc12/1
  [yyout3] -> reinterp_vacc13/1
  [xyoutre3] -> reinterp_vacc14/1
  [xyoutim3] -> reinterp_vacc15/1
  vacc0/1(valid) -> Terminator1/1
  Delay2/1 -> Delay17/1, Delay16/1, Delay1/1, Delay8/1, Delay3/1
  Delay17/1 -> [sync_out0]
  Delay16/1 -> [sync_out1]
  Delay8/1 -> [sync_out2]
  Delay3/1 -> [sync_out3]
  Delay1/1 -> [sync_out]
  gpio_tig_reg_1/1 -> Register22/1, Mux3/4
  gpio_tig_reg_3/1 -> bus_expand3/1(bus_in)
  ssg_bram_delay1/1 -> ssg_bram_delay2/1
  ssg_bram_delay2/1 -> SuperSlice/1(In)
  sg/1(sync_out) -> Subsytem/1(In1)
  Subsytem/1(Out1) -> [sync_gen_1]
  Subsytem/2(Out2) -> [sync_gen_0]
  Delay7/1 -> [a0]
  Delay9/1 -> [a1]
  Delay18/1 -> [a2]
  Delay19/1 -> [a3]
  Delay20/1 -> [a4]
  Delay22/1 -> [a5]
  Delay23/1 -> [a6]
  Delay24/1 -> [a7]
  Delay27/1 -> [b0]
  Delay28/1 -> [b1]
  Delay31/1 -> [b2]
  Delay33/1 -> [b3]
  Delay34/1 -> [b4]
  Delay35/1 -> [b5]
  Delay36/1 -> [b6]
  Delay37/1 -> [b7]
  [xy_vaccre3] -> cram4/1(In1)
  [xy_vaccim3] -> cram4/2(In2)
  [xx_vacc3] -> cram4/3(In3)
  [yy_vacc3] -> cram4/4(In4)
  [xy_vaccre2] -> cram4/5(In5)
  [xy_vaccim2] -> cram4/6(In6)
  [xx_vacc2] -> cram4/7(In7)
  [yy_vacc2] -> cram4/8(In8)
  [xy_vaccre1] -> cram4/9(In9)
  [xy_vaccim1] -> cram4/10(In10)
  [xx_vacc1] -> cram4/11(In11)
  [yy_vacc1] -> cram4/12(In12)
  [xy_vaccre0] -> cram4/13(In13)
  [xy_vaccim0] -> cram4/14(In14)
  [xx_vacc0] -> cram4/15(In15)
  [yy_vacc0] -> cram4/16(In16)
  sg/2(pps_out) -> Subsystem/1(In2)
  Subsystem/1(Out1) -> [master_rst1]
  Subsystem/2(Out2) -> [master_rst2]
  Subsystem/3(Out3) -> [master_rst3]
  Subsystem/4(Out4) -> [master_rst4]
  Slice1/1 -> [xx_vacc0]
  Slice11/1 -> [yy_vacc0]
  Slice12/1 -> [xy_vaccre0]
  Slice16/1 -> [xy_vaccim0]
  Slice17/1 -> [xx_vacc1]
  Slice23/1 -> [yy_vacc1]
  Slice24/1 -> [xy_vaccre1]
  Slice31/1 -> [xy_vaccim1]
  Slice32/1 -> [xx_vacc2]
  Slice33/1 -> [yy_vacc2]
  Slice34/1 -> [xy_vaccre2]
  Slice35/1 -> [xy_vaccim2]
  Slice36/1 -> [xx_vacc3]
  Slice37/1 -> [yy_vacc3]
  Slice38/1 -> [xy_vaccre3]
  Slice39/1 -> [xy_vaccim3]
  fft_wideband_real1/5(dout3) -> Register2/1
  fft_wideband_real1/4(dout2) -> Register1/1
  pfb_fir_real1/2(out0) -> fft_wideband_real1/3(din_0)
  pfb_fir_real1/3(out1) -> fft_wideband_real1/4(din_1)
  pfb_fir_real1/4(out2) -> fft_wideband_real1/5(din_2)
  pfb_fir_real1/5(out3) -> fft_wideband_real1/6(din_3)
  pfb_fir_real1/6(out4) -> fft_wideband_real1/7(din_4)
  pfb_fir_real1/7(out5) -> fft_wideband_real1/8(din_5)
  pfb_fir_real1/8(out6) -> fft_wideband_real1/9(din_6)
  pfb_fir_real1/9(out7) -> fft_wideband_real1/10(din_7)
  reinterp_vacc/1 -> vacc0/3(din_1_1)
  reinterp_vacc1/1 -> vacc0/4(din_2_1)
  reinterp_vacc2/1 -> vacc0/5(din_3_1)
  reinterp_vacc3/1 -> vacc0/6(din_4_1)
  reinterp_vacc4/1 -> vacc1/3(din_1_1)
  reinterp_vacc5/1 -> vacc1/4(din_2_1)
  reinterp_vacc6/1 -> vacc1/5(din_3_1)
  reinterp_vacc7/1 -> vacc1/6(din_4_1)
  reinterp_vacc8/1 -> vacc2/3(din_1_1)
  reinterp_vacc9/1 -> vacc2/4(din_2_1)
  reinterp_vacc10/1 -> vacc2/5(din_3_1)
  reinterp_vacc11/1 -> vacc2/6(din_4_1)
  reinterp_vacc12/1 -> vacc3/3(din_1_1)
  reinterp_vacc13/1 -> vacc3/4(din_2_1)
  reinterp_vacc14/1 -> vacc3/5(din_3_1)
  reinterp_vacc15/1 -> vacc3/6(din_4_1)
